simulink model "leda_actual_1s"  (Simulink 7.4)
settings: Solver=ode45  StartTime=0.0  StopTime=50000  RelTol=1e-3
undefined symbols (not defined in the file): pfb_coeff_gen_calc
libraries: xbsIndex_r4 x5755, casper_library_misc x176, casper_library_delays x48, xps_library x39, xbsReplacements_r4 x4, casper_library_ffts_twiddle x2, simulink x1
(part 8 of 84)

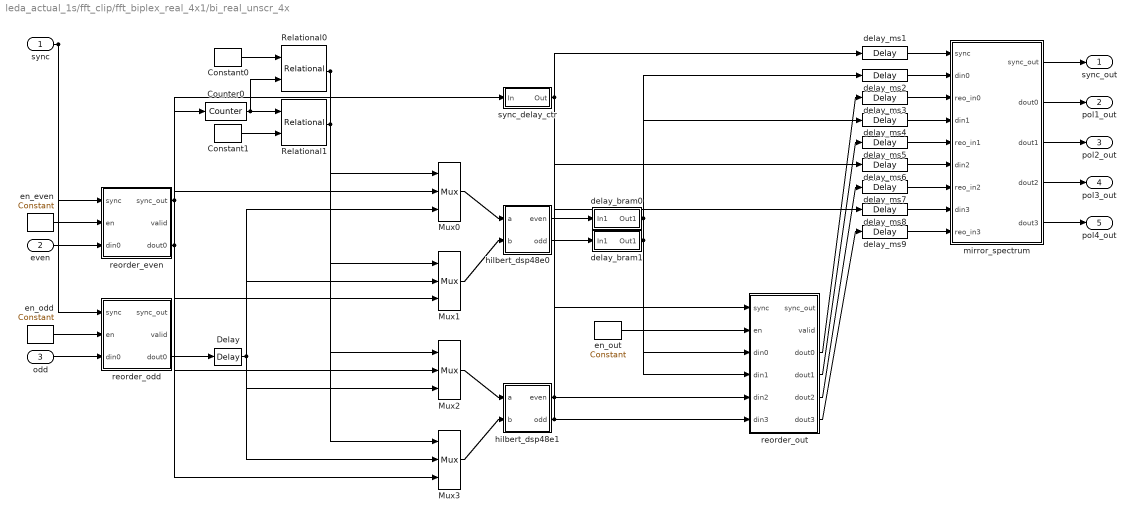
== system leda_actual_1s/fft_clip/fft_biplex_real_4x1/bi_real_unscr_4x ==
  sync : Inport
  even : Inport  Port=2
  odd : Inport  Port=3
  Constant0 : xbsIndex_r4/Constant  arith_type=Unsigned  const=2^(FFTSize-1)  n_bits=FFTSize  bin_pt=0  explicit_period=on  period=1  equ=P=C  opselect=C  inp2=PCIN>>17  opr=+  inp1=P  carry=CIN  dbl_ovrd=off  block_type=constant
      settings: block_version=11.4
  Constant1 : xbsIndex_r4/Constant  arith_type=Unsigned  const=0  n_bits=FFTSize  bin_pt=0  explicit_period=on  period=1  equ=P=C  opselect=C  inp2=PCIN>>17  opr=+  inp1=P  carry=CIN  dbl_ovrd=off  block_type=constant
      settings: block_version=11.4
  Counter0 : xbsIndex_r4/Counter  cnt_type=Free Running  cnt_to=Inf  operation=Up  start_count=0  cnt_by_val=1  arith_type=Unsigned  n_bits=FFTSize  bin_pt=0  load_pin=off  rst=on  en=off  explicit_period=on  period=1  dbl_ovrd=off  use_behavioral_HDL=off  implementation=Fabric  block_type=counter
      settings: block_version=11.4
  Delay : xbsIndex_r4/Delay  en=off  latency=1  dbl_ovrd=off  reg_retiming=off  block_type=delay
      settings: block_version=11.4
  Mux{0..3} (4 alike) : xbsIndex_r4/Mux  inputs=2  en=off  latency=1  precision=Full  arith_type=Unsigned  n_bits=16  bin_pt=14  quantization=Truncate  overflow=Wrap  dbl_ovrd=off  block_type=mux
      settings: block_version=11.4
  Relational0 : xbsIndex_r4/Relational  mode=a=b  en=off  latency=0  dbl_ovrd=off  block_type=relational
  Relational1 : xbsIndex_r4/Relational  mode=a=b  en=off  latency=0  dbl_ovrd=off  block_type=relational
  delay_bram0 : Subsystem  AncestorBlock=casper_library_delays/delay_bram  mask(delay_bram){DelayLen=2^(FFTSize-1), bram_latency=bram_latency, use_dsp48=off}
      mask prompts: DelayLen "Delay By:"; bram_latency "BRAM Latency"; use_dsp48 "Use DSP48"
      mask help: A delay block that uses BRAM for its storage.
      mask init:
      | if (DelayLen <= bram_latency)
      | 	error('delay value must be greater than BRAM Latency');
      | end
      | BitWidth = max(ceil(log2(DelayLen)), 2);
      | if strcmp(use_dsp48,'on'),
      |     set_param([gcb,'/Counter'], 'use_behavioral_HDL', 'off', ...
      |         'use_rpm', 'on', 'implementation', 'DSP48');
      | else, %default counter settings
      |         set_param([gcb,'/Counter'], 'use_behavioral_HDL', 'off', ...
      |         'use_rpm', 'off', 'implementation', 'Fabric');
      | end
      mask Help: eval('xlWeb(''http://casper.berkeley.edu/wiki/Delay_bram'')')
  delay_bram1 : Subsystem  AncestorBlock=casper_library_delays/delay_bram  mask(delay_bram){DelayLen=2^(FFTSize-1), bram_latency=bram_latency, use_dsp48=off}
      mask prompts: DelayLen "Delay By:"; bram_latency "BRAM Latency"; use_dsp48 "Use DSP48"
      mask help: A delay block that uses BRAM for its storage.
      mask init: same as leda_actual_1s/fft_clip/fft_biplex_real_4x1/bi_real_unscr_4x/delay_bram0
      mask Help: eval('xlWeb(''http://casper.berkeley.edu/wiki/Delay_bram'')')
  delay_ms{1..9} (9 alike) : xbsIndex_r4/Delay  en=off  latency=1  dbl_ovrd=off  reg_retiming=off  block_type=delay
      settings: block_version=11.4
  en_even : xbsIndex_r4/Constant  arith_type=Boolean  const=1  n_bits=1  bin_pt=0  explicit_period=on  period=1  equ=P=C  opselect=C  inp2=PCIN>>17  opr=+  inp1=P  carry=CIN  dbl_ovrd=off  block_type=constant
      settings: block_version=11.4
  en_odd : xbsIndex_r4/Constant  arith_type=Boolean  const=1  n_bits=1  bin_pt=0  explicit_period=on  period=1  equ=P=C  opselect=C  inp2=PCIN>>17  opr=+  inp1=P  carry=CIN  dbl_ovrd=off  block_type=constant
      settings: block_version=11.4
  en_out : xbsIndex_r4/Constant  arith_type=Boolean  const=1  n_bits=1  bin_pt=0  explicit_period=on  period=1  equ=P=C  opselect=C  inp2=PCIN>>17  opr=+  inp1=P  carry=CIN  dbl_ovrd=off  block_type=constant
      settings: block_version=11.4
  hilbert_dsp48e0 : Subsystem  AncestorBlock=casper_library_ffts_internal/hilbert_dsp48e  mask(sincos_dsp48e){BitWidth=n_bits, conv_latency=conv_latency}
      mask prompts: BitWidth "Bit Wdith"; conv_latency "Convert Latency"
      mask help:
      | FFT internal block.
      |
      | Computes (a+b*)/2 and (a-b*)/2i.
  hilbert_dsp48e1 : Subsystem  AncestorBlock=casper_library_ffts_internal/hilbert_dsp48e  mask(sincos_dsp48e){BitWidth=n_bits, conv_latency=conv_latency}
      mask prompts: BitWidth "Bit Wdith"; conv_latency "Convert Latency"
      mask help:
      | FFT internal block.
      |
      | Computes (a+b*)/2 and (a-b*)/2i.
  mirror_spectrum : Subsystem  AncestorBlock=casper_library_ffts_internal/mirror_spectrum  mask(mirror_spectrum){FFTSize=13, input_bitwidth=18, bram_latency=3, negate_latency=1}
      mask prompts: FFTSize "Size of FFT (2^?)"; input_bitwidth "Input Bit Width"; bram_latency "BRAM Latency"; negate_latency "Negate Latency"
      mask help: FFT internal block.
      mask TabNameString: Basic,Basic,Latency,Latency
  reorder_even : Subsystem  AncestorBlock=casper_library_reorder/reorder  mask(reorder){map=[0 2048 1024 3072 512 2560 1536 3584 256 2304 1280 3328 768 2816 1792 3840 128 2176 1152 3200 640 2688 1664 3712 384 2432 1408 3456 896 2944 1920 3968 64 2112 1088 3136 576 2624 1600 3648 320 2368 1344 3392 832 2880 1856 3904 192 2240 1216 3264 704 2752 1728 3776 448 2496 1472 3520 960 3008 1984 4032 32 2080 1056 3104 544 2592 1568 3616 288 2336 1312 3360 800 2848 1824 3872 160 2208 1184 3232 672 2720 1696 3744 416 2464 1440 3488 928 2976 1952 4000 96 2144 1120 3168 608 2656 1632 3680 352 2400 1376 3424 864 2912 1888 3936 224 2272 1248 3296 736 2784 1760 3808 480 2528 1504 3552 992 3040 2016 4064 16 2064 1040 3088 528 2576 1552 3600 272 2320 1296 3344 784 2832 1808 3856 144 2192 1168 3216 656 2704 1680 3728 400 2448 1424 3472 912 2960 1936 3984 80 2128 1104 3152 592 2640 1616 3664 336 2384 1360 3408 848 2896 1872 3920 208 2256 1232 3280 720 2768 1744 3792 464 2512 1488 3536 976 3024 2000 4048 48 2096 1072 3120 560 2608 1584 3632 304 2352 1328 3376 816 2864 1840 3888 176 2224 1200 3248 688 2736 1712 3760 432 2480 1456 3504 944 2992 1968 4016 112 2160 1136 3184 624 2672 1648 3696 368 2416 1392 3440 880 2928 1904 3952 240 2288 1264 3312 752 2800 1776 3824 496 2544 1520 3568 1008 3056 2032 4080 8 2056 1032 3080 520 2568 1544 3592 264 2312 1288 3336 776 2824 1800 3848 136 2184 1160 3208 648 2696 1672 3720 392 2440 1416 3464 904 2952 1928 3976 72 2120 1096 3144 584 2632 1608 3656 328 2376 1352 3400 840 2888 1864 3912 200 2248 1224 3272 712 2760 1736 3784 456 2504 1480 3528 968 3016 1992 4040 40 2088 1064 3112 552 2600 1576 3624 296 2344 1320 3368 808 2856 1832 3880 168 2216 1192 3240 680 2728 1704 3752 424 2472 1448 3496 936 2984 1960 4008 104 2152 1128 3176 616 2664 1640 3688 360 2408 1384 3432 872 2920 1896 3944 232 2280 1256 3304 744 2792 1768 3816 488 2536 1512 3560 1000 3048 2024 4072 24 2072 1048 3096 536 2584 1560 3608 280 2328 1304 3352 792 2840 1816 3864 152 2200 1176 3224 664 2712 1688 3736 408 2456 1432 3480 920 2968 1944 3992 88 2136 1112 3160 600 2648 1624 3672 344 2392 1368 3416 856 2904 1880 3928 216 2264 1240 3288 728 2776 1752 3800 472 2520 1496 3544 984 3032 2008 4056 56 2104 1080 3128 568 2616 1592 3640 312 2360 1336 3384 824 2872 1848 3896 184 2232 1208 3256 696 2744 1720 3768 440 2488 1464 3512 952 3000 1976 4024 120 2168 1144 3192 632 2680 1656 3704 376 2424 1400 3448 888 2936 1912 3960 248 2296 1272 3320 760 2808 1784 3832 504 2552 1528 3576 1016 3064 2040 4088 4 2052 1028 3076 516 2564 1540 3588 260 2308 1284 3332 772 2820 1796 3844 132 2180 1156 3204 644 2692 1668 3716 388 2436 1412 3460 900 2948 1924 3972 68 2116 1092 3140 580 2628 1604 3652 324 2372 1348 3396 836 2884 1860 3908 196 2244 1220 3268 708 2756 1732 3780 452 2500 1476 3524 964 3012 1988 4036 36 2084 1060 3108 548 2596 1572 3620 292 2340 1316 3364 804 2852 1828 3876 164 2212 1188 3236 676 2724 1700 3748 420 2468 1444 3492 932 2980 1956 4004 100 2148 1124 3172 612 2660 1636 3684 356 2404 1380 3428 868 2916 1892 3940 228 2276 1252 3300 740 2788 1764 3812 484 2532 1508 3556 996 3044 2020 4068 20 2068 1044 3092 532 2580 1556 3604 276 2324 1300 3348 788 2836 1812 3860 148 2196 1172 3220 660 2708 1684 3732 404 2452 1428 3476 916 2964 1940 3988 84 2132 1108 3156 596 2644 1620 3668 340 2388 1364 3412 852 2900 1876 3924 212 2260 1236 3284 724 2772 1748 3796 468 2516 1492 3540 980 3028 2004 4052 52 2100 1076 3124 564 2612 1588 3636 308 2356 1332 3380 820 2868 1844 3892 180 2228 1204 3252 692 2740 1716 3764 436 2484 1460 3508 948 2996 1972 4020 116 2164 1140 3188 628 2676 1652 3700 372 2420 1396 3444 884 2932 1908 3956 244 2292 1268 3316 756 2804 1780 3828 500 2548 1524 3572 1012 3060 2036 4084 12 2060 1036 3084 524 2572 1548 3596 268 2316 1292 3340 780 2828 1804 3852 140 2188 1164 3212 652 2700 1676 3724 396 2444 1420 3468 908 2956 1932 3980 76 2124 1100 3148 588 2636 1612 3660 332 2380 1356 3404 844 2892 1868 3916 204 2252 1228 3276 716 2764 1740 3788 460 2508 1484 3532 972 3020 1996 4044 44 2092 1068 3116 556 2604 1580 3628 300 2348 1324 3372 812 2860 ...(+15373 chars)], n_inputs=1, bram_latency=bram_latency, map_latency=0, double_buffer=0, bram_map=off}
      mask prompts: map "Output Order:"; n_inputs "Number of inputs"; bram_latency "BRAM Latency"; map_latency "Map Latency"; double_buffer "Double Buffer"; bram_map "Use BlockRAM for Map"
      mask init:
      | reorder_init(gcb, ...
      |     'map', map, ...
      |     'n_inputs', n_inputs, ...
      |     'bram_latency', bram_latency, ...
      |     'map_latency', map_latency, ...
      |     'double_buffer', double_buffer, ...
      |     'bram_map', bram_map);
      settings: AttributesFormatString=order=2
      mask Help: eval('xlWeb(''http://casper.berkeley.edu/wiki/Reorder'')')
  reorder_odd : Subsystem  AncestorBlock=casper_library_reorder/reorder  mask(reorder){map=[4095 2047 3071 1023 3583 1535 2559 511 3839 1791 2815 767 3327 1279 2303 255 3967 1919 2943 895 3455 1407 2431 383 3711 1663 2687 639 3199 1151 2175 127 4031 1983 3007 959 3519 1471 2495 447 3775 1727 2751 703 3263 1215 2239 191 3903 1855 2879 831 3391 1343 2367 319 3647 1599 2623 575 3135 1087 2111 63 4063 2015 3039 991 3551 1503 2527 479 3807 1759 2783 735 3295 1247 2271 223 3935 1887 2911 863 3423 1375 2399 351 3679 1631 2655 607 3167 1119 2143 95 3999 1951 2975 927 3487 1439 2463 415 3743 1695 2719 671 3231 1183 2207 159 3871 1823 2847 799 3359 1311 2335 287 3615 1567 2591 543 3103 1055 2079 31 4079 2031 3055 1007 3567 1519 2543 495 3823 1775 2799 751 3311 1263 2287 239 3951 1903 2927 879 3439 1391 2415 367 3695 1647 2671 623 3183 1135 2159 111 4015 1967 2991 943 3503 1455 2479 431 3759 1711 2735 687 3247 1199 2223 175 3887 1839 2863 815 3375 1327 2351 303 3631 1583 2607 559 3119 1071 2095 47 4047 1999 3023 975 3535 1487 2511 463 3791 1743 2767 719 3279 1231 2255 207 3919 1871 2895 847 3407 1359 2383 335 3663 1615 2639 591 3151 1103 2127 79 3983 1935 2959 911 3471 1423 2447 399 3727 1679 2703 655 3215 1167 2191 143 3855 1807 2831 783 3343 1295 2319 271 3599 1551 2575 527 3087 1039 2063 15 4087 2039 3063 1015 3575 1527 2551 503 3831 1783 2807 759 3319 1271 2295 247 3959 1911 2935 887 3447 1399 2423 375 3703 1655 2679 631 3191 1143 2167 119 4023 1975 2999 951 3511 1463 2487 439 3767 1719 2743 695 3255 1207 2231 183 3895 1847 2871 823 3383 1335 2359 311 3639 1591 2615 567 3127 1079 2103 55 4055 2007 3031 983 3543 1495 2519 471 3799 1751 2775 727 3287 1239 2263 215 3927 1879 2903 855 3415 1367 2391 343 3671 1623 2647 599 3159 1111 2135 87 3991 1943 2967 919 3479 1431 2455 407 3735 1687 2711 663 3223 1175 2199 151 3863 1815 2839 791 3351 1303 2327 279 3607 1559 2583 535 3095 1047 2071 23 4071 2023 3047 999 3559 1511 2535 487 3815 1767 2791 743 3303 1255 2279 231 3943 1895 2919 871 3431 1383 2407 359 3687 1639 2663 615 3175 1127 2151 103 4007 1959 2983 935 3495 1447 2471 423 3751 1703 2727 679 3239 1191 2215 167 3879 1831 2855 807 3367 1319 2343 295 3623 1575 2599 551 3111 1063 2087 39 4039 1991 3015 967 3527 1479 2503 455 3783 1735 2759 711 3271 1223 2247 199 3911 1863 2887 839 3399 1351 2375 327 3655 1607 2631 583 3143 1095 2119 71 3975 1927 2951 903 3463 1415 2439 391 3719 1671 2695 647 3207 1159 2183 135 3847 1799 2823 775 3335 1287 2311 263 3591 1543 2567 519 3079 1031 2055 7 4091 2043 3067 1019 3579 1531 2555 507 3835 1787 2811 763 3323 1275 2299 251 3963 1915 2939 891 3451 1403 2427 379 3707 1659 2683 635 3195 1147 2171 123 4027 1979 3003 955 3515 1467 2491 443 3771 1723 2747 699 3259 1211 2235 187 3899 1851 2875 827 3387 1339 2363 315 3643 1595 2619 571 3131 1083 2107 59 4059 2011 3035 987 3547 1499 2523 475 3803 1755 2779 731 3291 1243 2267 219 3931 1883 2907 859 3419 1371 2395 347 3675 1627 2651 603 3163 1115 2139 91 3995 1947 2971 923 3483 1435 2459 411 3739 1691 2715 667 3227 1179 2203 155 3867 1819 2843 795 3355 1307 2331 283 3611 1563 2587 539 3099 1051 2075 27 4075 2027 3051 1003 3563 1515 2539 491 3819 1771 2795 747 3307 1259 2283 235 3947 1899 2923 875 3435 1387 2411 363 3691 1643 2667 619 3179 1131 2155 107 4011 1963 2987 939 3499 1451 2475 427 3755 1707 2731 683 3243 1195 2219 171 3883 1835 2859 811 3371 1323 2347 299 3627 1579 2603 555 3115 1067 2091 43 4043 1995 3019 971 3531 1483 2507 459 3787 1739 2763 715 3275 1227 2251 203 3915 1867 2891 843 3403 1355 2379 331 3659 1611 2635 587 3147 1099 2123 75 3979 1931 2955 907 3467 1419 2443 395 3723 1675 2699 651 3211 1163 2187 139 3851 1803 2827 779 3339 1291 2315 267 3595 1547 2571 523 3083 1035 2059 11 4083 2035 3059 1011 3571 1523 2547 499 3827 1779 2803 755 3315 1267 2291 243 3955 1907 2931 883 3443 1395 2419 371 3699 1651 2675 627 3187 1139 2163 115 4019 1971 2995 947 3507 1459 2483 435 3763 1715 2739 691 3251 1203 2227 179 3891 1843 2867 819 3379 1331 2355 307 3635 1587 2611 563 3123 1075 2099 51 4051 2003 3027 979 3539 1491 2515 467 3795 1747 2771 723 ...(+15375 chars)], n_inputs=1, bram_latency=bram_latency, map_latency=0, double_buffer=0, bram_map=off}
      mask prompts: map "Output Order:"; n_inputs "Number of inputs"; bram_latency "BRAM Latency"; map_latency "Map Latency"; double_buffer "Double Buffer"; bram_map "Use BlockRAM for Map"
      mask init: same as leda_actual_1s/fft_clip/fft_biplex_real_4x1/bi_real_unscr_4x/reorder_even
      settings: AttributesFormatString=order=2
      mask Help: eval('xlWeb(''http://casper.berkeley.edu/wiki/Reorder'')')
  reorder_out : Subsystem  AncestorBlock=casper_library_reorder/reorder  mask(reorder){map=[4095 4094 4093 4092 4091 4090 4089 4088 4087 4086 4085 4084 4083 4082 4081 4080 4079 4078 4077 4076 4075 4074 4073 4072 4071 4070 4069 4068 4067 4066 4065 4064 4063 4062 4061 4060 4059 4058 4057 4056 4055 4054 4053 4052 4051 4050 4049 4048 4047 4046 4045 4044 4043 4042 4041 4040 4039 4038 4037 4036 4035 4034 4033 4032 4031 4030 4029 4028 4027 4026 4025 4024 4023 4022 4021 4020 4019 4018 4017 4016 4015 4014 4013 4012 4011 4010 4009 4008 4007 4006 4005 4004 4003 4002 4001 4000 3999 3998 3997 3996 3995 3994 3993 3992 3991 3990 3989 3988 3987 3986 3985 3984 3983 3982 3981 3980 3979 3978 3977 3976 3975 3974 3973 3972 3971 3970 3969 3968 3967 3966 3965 3964 3963 3962 3961 3960 3959 3958 3957 3956 3955 3954 3953 3952 3951 3950 3949 3948 3947 3946 3945 3944 3943 3942 3941 3940 3939 3938 3937 3936 3935 3934 3933 3932 3931 3930 3929 3928 3927 3926 3925 3924 3923 3922 3921 3920 3919 3918 3917 3916 3915 3914 3913 3912 3911 3910 3909 3908 3907 3906 3905 3904 3903 3902 3901 3900 3899 3898 3897 3896 3895 3894 3893 3892 3891 3890 3889 3888 3887 3886 3885 3884 3883 3882 3881 3880 3879 3878 3877 3876 3875 3874 3873 3872 3871 3870 3869 3868 3867 3866 3865 3864 3863 3862 3861 3860 3859 3858 3857 3856 3855 3854 3853 3852 3851 3850 3849 3848 3847 3846 3845 3844 3843 3842 3841 3840 3839 3838 3837 3836 3835 3834 3833 3832 3831 3830 3829 3828 3827 3826 3825 3824 3823 3822 3821 3820 3819 3818 3817 3816 3815 3814 3813 3812 3811 3810 3809 3808 3807 3806 3805 3804 3803 3802 3801 3800 3799 3798 3797 3796 3795 3794 3793 3792 3791 3790 3789 3788 3787 3786 3785 3784 3783 3782 3781 3780 3779 3778 3777 3776 3775 3774 3773 3772 3771 3770 3769 3768 3767 3766 3765 3764 3763 3762 3761 3760 3759 3758 3757 3756 3755 3754 3753 3752 3751 3750 3749 3748 3747 3746 3745 3744 3743 3742 3741 3740 3739 3738 3737 3736 3735 3734 3733 3732 3731 3730 3729 3728 3727 3726 3725 3724 3723 3722 3721 3720 3719 3718 3717 3716 3715 3714 3713 3712 3711 3710 3709 3708 3707 3706 3705 3704 3703 3702 3701 3700 3699 3698 3697 3696 3695 3694 3693 3692 3691 3690 3689 3688 3687 3686 3685 3684 3683 3682 3681 3680 3679 3678 3677 3676 3675 3674 3673 3672 3671 3670 3669 3668 3667 3666 3665 3664 3663 3662 3661 3660 3659 3658 3657 3656 3655 3654 3653 3652 3651 3650 3649 3648 3647 3646 3645 3644 3643 3642 3641 3640 3639 3638 3637 3636 3635 3634 3633 3632 3631 3630 3629 3628 3627 3626 3625 3624 3623 3622 3621 3620 3619 3618 3617 3616 3615 3614 3613 3612 3611 3610 3609 3608 3607 3606 3605 3604 3603 3602 3601 3600 3599 3598 3597 3596 3595 3594 3593 3592 3591 3590 3589 3588 3587 3586 3585 3584 3583 3582 3581 3580 3579 3578 3577 3576 3575 3574 3573 3572 3571 3570 3569 3568 3567 3566 3565 3564 3563 3562 3561 3560 3559 3558 3557 3556 3555 3554 3553 3552 3551 3550 3549 3548 3547 3546 3545 3544 3543 3542 3541 3540 3539 3538 3537 3536 3535 3534 3533 3532 3531 3530 3529 3528 3527 3526 3525 3524 3523 3522 3521 3520 3519 3518 3517 3516 3515 3514 3513 3512 3511 3510 3509 3508 3507 3506 3505 3504 3503 3502 3501 3500 3499 3498 3497 3496 3495 3494 3493 3492 3491 3490 3489 3488 3487 3486 3485 3484 3483 3482 3481 3480 3479 3478 3477 3476 3475 3474 3473 3472 3471 3470 3469 3468 3467 3466 3465 3464 3463 3462 3461 3460 3459 3458 3457 3456 3455 3454 3453 3452 3451 3450 3449 3448 3447 3446 3445 3444 3443 3442 3441 3440 3439 3438 3437 3436 3435 3434 3433 3432 3431 3430 3429 3428 3427 3426 3425 3424 3423 3422 3421 3420 3419 3418 3417 3416 3415 3414 3413 3412 3411 3410 3409 3408 3407 3406 3405 3404 3403 3402 3401 3400 3399 3398 3397 3396 3395 3394 3393 3392 3391 3390 3389 3388 3387 3386 3385 3384 3383 3382 3381 3380 3379 3378 3377 3376 3375 3374 3373 3372 3371 3370 3369 3368 3367 3366 3365 3364 3363 3362 3361 3360 3359 3358 3357 3356 3355 3354 3353 3352 3351 3350 3349 3348 3347 3346 3345 3344 3343 3342 3341 3340 3339 3338 3337 3336 3335 3334 3333 3332 3331 3330 3329 3328 3327 3326 3325 3324 3323 3322 3321 3320 3319 3318 3317 3316 3315 3314 3313 3312 3311 3310 3309 3308 3307 3306 3305 3304 3303 3302 3301 3300 3299 3298 3297 ...(+15375 chars)], n_inputs=4, bram_latency=bram_latency, map_latency=0, double_buffer=0, bram_map=off}
      mask prompts: map "Output Order:"; n_inputs "Number of inputs"; bram_latency "BRAM Latency"; map_latency "Map Latency"; double_buffer "Double Buffer"; bram_map "Use BlockRAM for Map"
      mask init: same as leda_actual_1s/fft_clip/fft_biplex_real_4x1/bi_real_unscr_4x/reorder_even
      settings: AttributesFormatString=order=2
      mask Help: eval('xlWeb(''http://casper.berkeley.edu/wiki/Reorder'')')
  sync_delay_ctr : Subsystem  AncestorBlock=casper_library_delays/sync_delay  mask(sync_delay){DelayLen=2^(FFTSize-1) + add_latency + conv_latency + 1}
      mask prompts: DelayLen "Delay Length:"
      mask help: Delay an infrequent boolean pulse by the specified number of clocks.
      mask init:
      | BitWidth = max(2, ceil(log2(DelayLen+1)));
      | UseCounter = 1;
      | if DelayLen == 0,
      | 	UseCounter = 0;
      | end
      mask Help: eval('xlWeb(''http://casper.berkeley.edu/wiki/Sync_delay'')')
      mask Display: fprintf('Z^-%d', DelayLen)
  sync_out : Outport
  pol1_out : Outport  Port=2
  pol2_out : Outport  Port=3
  pol3_out : Outport  Port=4
  pol4_out : Outport  Port=5
  wires:
  sync/1 -> reorder_even/1(sync), reorder_odd/1(sync)
  en_even/1 -> reorder_even/2(en)
  even/1 -> reorder_even/3(din0)
  en_odd/1 -> reorder_odd/2(en)
  odd/1 -> reorder_odd/3(din0)
  reorder_odd/3(dout0) -> Delay/1
  reorder_even/1(sync_out) -> Counter0/1, sync_delay_ctr/1(In)
  Constant0/1 -> Relational0/1
  Counter0/1 -> Relational0/2, Relational1/1
  Constant1/1 -> Relational1/2
  Relational0/1 -> Mux0/1, Mux3/1
  Relational1/1 -> Mux1/1, Mux2/1
  reorder_even/3(dout0) -> Mux0/2, Mux1/3, Mux2/2, Mux3/3
  Delay/1 -> Mux0/3, Mux1/2, Mux2/3, Mux3/2
  Mux0/1 -> hilbert_dsp48e0/1(a)
  Mux1/1 -> hilbert_dsp48e0/2(b)
  Mux2/1 -> hilbert_dsp48e1/1(a)
  Mux3/1 -> hilbert_dsp48e1/2(b)
  hilbert_dsp48e0/1(even) -> delay_bram0/1(In1)
  hilbert_dsp48e0/2(odd) -> delay_bram1/1(In1)
  sync_delay_ctr/1(Out) -> reorder_out/1(sync), delay_ms1/1
  en_out/1 -> reorder_out/2(en)
  delay_bram0/1(Out1) -> reorder_out/3(din0), delay_ms2/1
  delay_bram1/1(Out1) -> reorder_out/4(din1), delay_ms4/1
  hilbert_dsp48e1/1(even) -> reorder_out/5(din2), delay_ms6/1
  hilbert_dsp48e1/2(odd) -> reorder_out/6(din3), delay_ms8/1
  reorder_out/3(dout0) -> delay_ms3/1
  reorder_out/4(dout1) -> delay_ms5/1
  reorder_out/5(dout2) -> delay_ms7/1
  reorder_out/6(dout3) -> delay_ms9/1
  delay_ms1/1 -> mirror_spectrum/1(sync)
  delay_ms2/1 -> mirror_spectrum/2(din0)
  delay_ms3/1 -> mirror_spectrum/3(reo_in0)
  delay_ms4/1 -> mirror_spectrum/4(din1)
  delay_ms5/1 -> mirror_spectrum/5(reo_in1)
  delay_ms6/1 -> mirror_spectrum/6(din2)
  delay_ms7/1 -> mirror_spectrum/7(reo_in2)
  delay_ms8/1 -> mirror_spectrum/8(din3)
  delay_ms9/1 -> mirror_spectrum/9(reo_in3)
  mirror_spectrum/1(sync_out) -> sync_out/1
  mirror_spectrum/2(dout0) -> pol1_out/1
  mirror_spectrum/3(dout1) -> pol2_out/1
  mirror_spectrum/4(dout2) -> pol3_out/1
  mirror_spectrum/5(dout3) -> pol4_out/1
